simulink model "CCMHfaMotor"  (R2022b)
settings: Solver=FixedStepDiscrete  StartTime=0.0  StopTime=10.0  FixedStep=0.01  RelTol=1e-3
model workspace (MAT data in the model file):
  HfaWakeUpFlag : Simulink.Signal (value not decoded)
  NvMBlockHfaMtrCalData : Simulink.Signal (value not decoded)
  NvMBlockHfaMtrCalDataInit : Simulink.Parameter (value not decoded)
symbols that are objects in the model workspace (value not decoded): NvMBlockHfaMtrCalDataInit
undefined symbols (not defined in the file or in its folder): ComChlType, DioChannelType, HFAChlType, LinFrameType, NetworkChlType, NvMBlockId, P_DioLevelType, P_LinIfSchHandleType, P_Std_ReturnType, PowerChlType
(part 4 of 4)

== system CCMHfaMotor/HfaNvmService ==
  HfaNvmService_ClerarNvmMotorData_sys : Subsystem

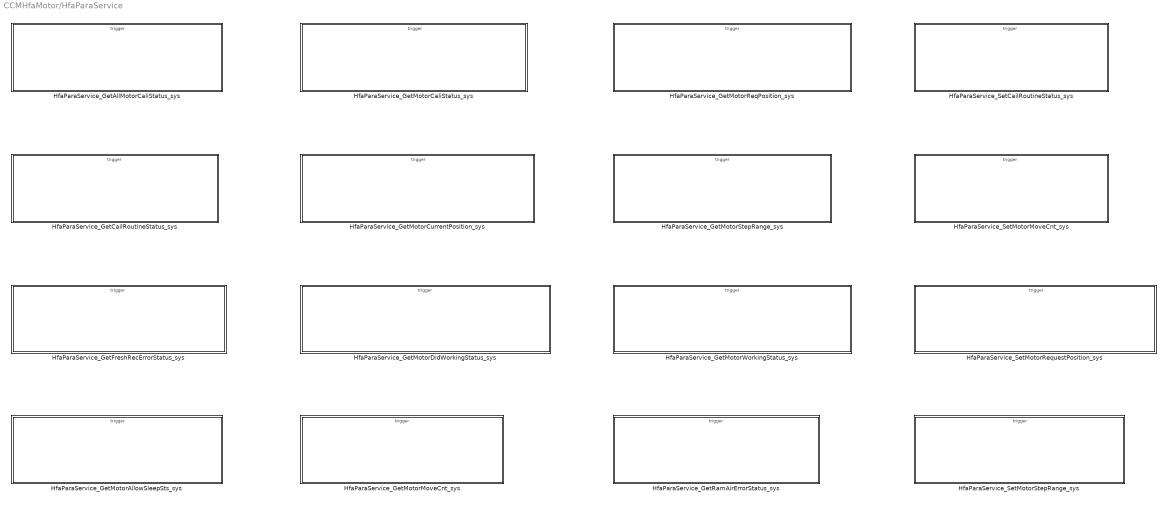
== system CCMHfaMotor/HfaParaService ==
  HfaParaService_GetAllMotorCaliStatus_sys : Subsystem
  HfaParaService_GetCailRoutineStatus_sys : Subsystem
  HfaParaService_GetFreshRecErrorStatus_sys : Subsystem
  HfaParaService_GetMotorAllowSleepSts_sys : Subsystem
  HfaParaService_GetMotorCaliStatus_sys : Subsystem
  HfaParaService_GetMotorCurrentPosition_sys : Subsystem
  HfaParaService_GetMotorDidWorkingStatus_sys : Subsystem
  HfaParaService_GetMotorMoveCnt_sys : Subsystem
  HfaParaService_GetMotorReqPosition_sys : Subsystem
  HfaParaService_GetMotorStepRange_sys : Subsystem
  HfaParaService_GetMotorWorkingStatus_sys : Subsystem
  HfaParaService_GetRamAirErrorStatus_sys : Subsystem
  HfaParaService_SetCailRoutineStatus_sys : Subsystem
  HfaParaService_SetMotorMoveCnt_sys : Subsystem
  HfaParaService_SetMotorRequestPosition_sys : Subsystem
  HfaParaService_SetMotorStepRange_sys : Subsystem

== system CCMHfaMotor/HfaRIDService ==
  HfaRIDService_GetAutoAddreRoutineSts_sys : Subsystem
  HfaRIDService_GetMotorAutoAddreSts_sys : Subsystem
  HfaRIDService_SetAutoAddreRoutineSts_sys : Subsystem
  HfaRIDService_SetMotorAutoAddre_sys : Subsystem

== system CCMHfaMotor/HfaCaliService/HfaCaliService_SetAllCalibrate_sys ==
  function : TriggerPort  TriggerType=function-call  IsSimulinkFunction=on  FunctionName=HfaCaliService_SetAllCalibrate  VariantControl=(inherit)
  Ground_1 : Ground
  PA_NvMBlockHfaMtrCalDataInit : Constant  Value=NvMBlockHfaMtrCalDataInit
  Terminator_1 : Terminator
  ERR : ArgOut  OutDataTypeStr=Std_ReturnType  ArgumentName=ERR
  wires:
  PA_NvMBlockHfaMtrCalDataInit/1 -> Terminator_1/1
  Ground_1/1 -> ERR/1

== system CCMHfaMotor/HfaCaliService/HfaCaliService_SetAllParaInit_sys ==
  function : TriggerPort  TriggerType=function-call  IsSimulinkFunction=on  FunctionName=HfaCaliService_SetAllParaInit  VariantControl=(inherit)
  Ground_1 : Ground
  PA_NvMBlockHfaMtrCalDataInit : Constant  Value=NvMBlockHfaMtrCalDataInit
  Terminator_1 : Terminator
  ERR : ArgOut  OutDataTypeStr=Std_ReturnType  ArgumentName=ERR
  wires:
  PA_NvMBlockHfaMtrCalDataInit/1 -> Terminator_1/1
  Ground_1/1 -> ERR/1

== system CCMHfaMotor/HfaCaliService/HfaCaliService_SetMotorCalibrate_sys ==
  function : TriggerPort  TriggerType=function-call  IsSimulinkFunction=on  FunctionName=HfaCaliService_SetMotorCalibrate  VariantControl=(inherit)
  Ground_1 : Ground
  PA_NvMBlockHfaMtrCalDataInit : Constant  Value=NvMBlockHfaMtrCalDataInit
  Terminator_1 : Terminator
  Terminator_2 : Terminator
  Channel : ArgIn  OutDataTypeStr=Enum: HFAChlType  ArgumentName=Channel
  ERR : ArgOut  OutDataTypeStr=Std_ReturnType  ArgumentName=ERR
  wires:
  PA_NvMBlockHfaMtrCalDataInit/1 -> Terminator_1/1
  Channel/1 -> Terminator_2/1
  Ground_1/1 -> ERR/1

== system CCMHfaMotor/HfaCaliService/HfaCaliService_SetMotorParaInit_sys ==
  function : TriggerPort  TriggerType=function-call  IsSimulinkFunction=on  FunctionName=HfaCaliService_SetMotorParaInit  VariantControl=(inherit)
  Ground_1 : Ground
  PA_NvMBlockHfaMtrCalDataInit : Constant  Value=NvMBlockHfaMtrCalDataInit
  Terminator_1 : Terminator
  Terminator_2 : Terminator
  Channel : ArgIn  OutDataTypeStr=Enum: HFAChlType  ArgumentName=Channel
  ERR : ArgOut  OutDataTypeStr=Std_ReturnType  ArgumentName=ERR
  wires:
  PA_NvMBlockHfaMtrCalDataInit/1 -> Terminator_1/1
  Channel/1 -> Terminator_2/1
  Ground_1/1 -> ERR/1

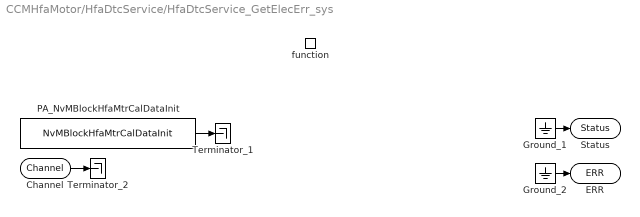
== system CCMHfaMotor/HfaDtcService/HfaDtcService_GetElecErr_sys ==
  function : TriggerPort  TriggerType=function-call  IsSimulinkFunction=on  FunctionName=HfaDtcService_GetElecErr  VariantControl=(inherit)
  Ground_1 : Ground
  Ground_2 : Ground
  PA_NvMBlockHfaMtrCalDataInit : Constant  Value=NvMBlockHfaMtrCalDataInit
  Terminator_1 : Terminator
  Terminator_2 : Terminator
  Channel : ArgIn  OutDataTypeStr=Enum: HFAChlType  ArgumentName=Channel
  Status : ArgOut  OutDataTypeStr=UInt8  ArgumentName=Status
  ERR : ArgOut  Port=2  OutDataTypeStr=Std_ReturnType  ArgumentName=ERR
  wires:
  PA_NvMBlockHfaMtrCalDataInit/1 -> Terminator_1/1
  Channel/1 -> Terminator_2/1
  Ground_1/1 -> Status/1
  Ground_2/1 -> ERR/1

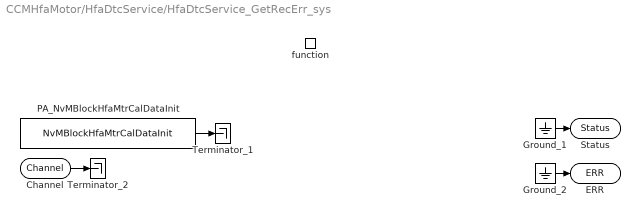
== system CCMHfaMotor/HfaDtcService/HfaDtcService_GetRecErr_sys ==
  function : TriggerPort  TriggerType=function-call  IsSimulinkFunction=on  FunctionName=HfaDtcService_GetRecErr  VariantControl=(inherit)
  Ground_1 : Ground
  Ground_2 : Ground
  PA_NvMBlockHfaMtrCalDataInit : Constant  Value=NvMBlockHfaMtrCalDataInit
  Terminator_1 : Terminator
  Terminator_2 : Terminator
  Channel : ArgIn  OutDataTypeStr=Enum: HFAChlType  ArgumentName=Channel
  Status : ArgOut  OutDataTypeStr=UInt8  ArgumentName=Status
  ERR : ArgOut  Port=2  OutDataTypeStr=Std_ReturnType  ArgumentName=ERR
  wires:
  PA_NvMBlockHfaMtrCalDataInit/1 -> Terminator_1/1
  Channel/1 -> Terminator_2/1
  Ground_1/1 -> Status/1
  Ground_2/1 -> ERR/1

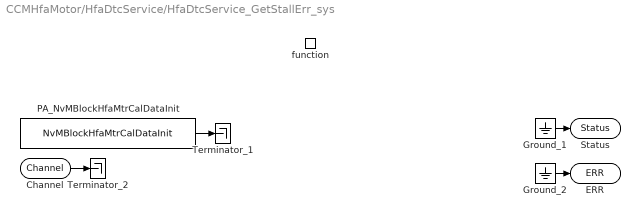
== system CCMHfaMotor/HfaDtcService/HfaDtcService_GetStallErr_sys ==
  function : TriggerPort  TriggerType=function-call  IsSimulinkFunction=on  FunctionName=HfaDtcService_GetStallErr  VariantControl=(inherit)
  Ground_1 : Ground
  Ground_2 : Ground
  PA_NvMBlockHfaMtrCalDataInit : Constant  Value=NvMBlockHfaMtrCalDataInit
  Terminator_1 : Terminator
  Terminator_2 : Terminator
  Channel : ArgIn  OutDataTypeStr=Enum: HFAChlType  ArgumentName=Channel
  Status : ArgOut  OutDataTypeStr=UInt8  ArgumentName=Status
  ERR : ArgOut  Port=2  OutDataTypeStr=Std_ReturnType  ArgumentName=ERR
  wires:
  PA_NvMBlockHfaMtrCalDataInit/1 -> Terminator_1/1
  Channel/1 -> Terminator_2/1
  Ground_1/1 -> Status/1
  Ground_2/1 -> ERR/1

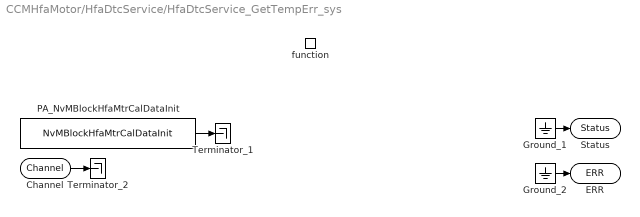
== system CCMHfaMotor/HfaDtcService/HfaDtcService_GetTempErr_sys ==
  function : TriggerPort  TriggerType=function-call  IsSimulinkFunction=on  FunctionName=HfaDtcService_GetTempErr  VariantControl=(inherit)
  Ground_1 : Ground
  Ground_2 : Ground
  PA_NvMBlockHfaMtrCalDataInit : Constant  Value=NvMBlockHfaMtrCalDataInit
  Terminator_1 : Terminator
  Terminator_2 : Terminator
  Channel : ArgIn  OutDataTypeStr=Enum: HFAChlType  ArgumentName=Channel
  Status : ArgOut  OutDataTypeStr=UInt8  ArgumentName=Status
  ERR : ArgOut  Port=2  OutDataTypeStr=Std_ReturnType  ArgumentName=ERR
  wires:
  PA_NvMBlockHfaMtrCalDataInit/1 -> Terminator_1/1
  Channel/1 -> Terminator_2/1
  Ground_1/1 -> Status/1
  Ground_2/1 -> ERR/1

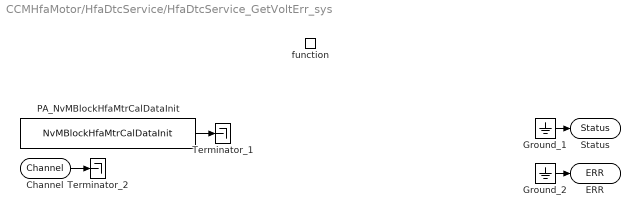
== system CCMHfaMotor/HfaDtcService/HfaDtcService_GetVoltErr_sys ==
  function : TriggerPort  TriggerType=function-call  IsSimulinkFunction=on  FunctionName=HfaDtcService_GetVoltErr  VariantControl=(inherit)
  Ground_1 : Ground
  Ground_2 : Ground
  PA_NvMBlockHfaMtrCalDataInit : Constant  Value=NvMBlockHfaMtrCalDataInit
  Terminator_1 : Terminator
  Terminator_2 : Terminator
  Channel : ArgIn  OutDataTypeStr=Enum: HFAChlType  ArgumentName=Channel
  Status : ArgOut  OutDataTypeStr=UInt8  ArgumentName=Status
  ERR : ArgOut  Port=2  OutDataTypeStr=Std_ReturnType  ArgumentName=ERR
  wires:
  PA_NvMBlockHfaMtrCalDataInit/1 -> Terminator_1/1
  Channel/1 -> Terminator_2/1
  Ground_1/1 -> Status/1
  Ground_2/1 -> ERR/1

== system CCMHfaMotor/HfaIOCtrlService/HfaIOCtrlService_FreezeMotorCurrentPosition_sys ==
  function : TriggerPort  TriggerType=function-call  IsSimulinkFunction=on  FunctionName=HfaIOCtrlService_FreezeMotorCurrentPosition  VariantControl=(inherit)
  Ground_1 : Ground
  PA_NvMBlockHfaMtrCalDataInit : Constant  Value=NvMBlockHfaMtrCalDataInit
  Terminator_1 : Terminator
  Terminator_2 : Terminator
  Channel : ArgIn  OutDataTypeStr=Enum: HFAChlType  ArgumentName=Channel
  ERR : ArgOut  OutDataTypeStr=Std_ReturnType  ArgumentName=ERR
  wires:
  PA_NvMBlockHfaMtrCalDataInit/1 -> Terminator_1/1
  Channel/1 -> Terminator_2/1
  Ground_1/1 -> ERR/1

== system CCMHfaMotor/HfaIOCtrlService/HfaIOCtrlService_MotorReturnToECU_sys ==
  function : TriggerPort  TriggerType=function-call  IsSimulinkFunction=on  FunctionName=HfaIOCtrlService_MotorReturnToECU  VariantControl=(inherit)
  Ground_1 : Ground
  PA_NvMBlockHfaMtrCalDataInit : Constant  Value=NvMBlockHfaMtrCalDataInit
  Terminator_1 : Terminator
  Terminator_2 : Terminator
  Channel : ArgIn  OutDataTypeStr=Enum: HFAChlType  ArgumentName=Channel
  ERR : ArgOut  OutDataTypeStr=Std_ReturnType  ArgumentName=ERR
  wires:
  PA_NvMBlockHfaMtrCalDataInit/1 -> Terminator_1/1
  Channel/1 -> Terminator_2/1
  Ground_1/1 -> ERR/1

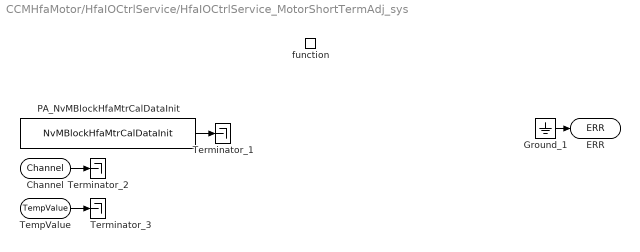
== system CCMHfaMotor/HfaIOCtrlService/HfaIOCtrlService_MotorShortTermAdj_sys ==
  function : TriggerPort  TriggerType=function-call  IsSimulinkFunction=on  FunctionName=HfaIOCtrlService_MotorShortTermAdj  VariantControl=(inherit)
  Ground_1 : Ground
  PA_NvMBlockHfaMtrCalDataInit : Constant  Value=NvMBlockHfaMtrCalDataInit
  Terminator_1 : Terminator
  Terminator_2 : Terminator
  Terminator_3 : Terminator
  Channel : ArgIn  OutDataTypeStr=Enum: HFAChlType  ArgumentName=Channel
  TempValue : ArgIn  Port=2  OutDataTypeStr=UInt16  ArgumentName=TempValue
  ERR : ArgOut  OutDataTypeStr=Std_ReturnType  ArgumentName=ERR
  wires:
  PA_NvMBlockHfaMtrCalDataInit/1 -> Terminator_1/1
  Channel/1 -> Terminator_2/1
  TempValue/1 -> Terminator_3/1
  Ground_1/1 -> ERR/1

== system CCMHfaMotor/HfaNvmService/HfaNvmService_ClerarNvmMotorData_sys ==
  function : TriggerPort  TriggerType=function-call  IsSimulinkFunction=on  FunctionName=HfaNvmService_ClerarNvmMotorData  VariantControl=(inherit)
  Ground_1 : Ground
  PA_NvMBlockHfaMtrCalDataInit : Constant  Value=NvMBlockHfaMtrCalDataInit
  Terminator_1 : Terminator
  ERR : ArgOut  OutDataTypeStr=Std_ReturnType  ArgumentName=ERR
  wires:
  PA_NvMBlockHfaMtrCalDataInit/1 -> Terminator_1/1
  Ground_1/1 -> ERR/1

== system CCMHfaMotor/HfaParaService/HfaParaService_GetAllMotorCaliStatus_sys ==
  function : TriggerPort  TriggerType=function-call  IsSimulinkFunction=on  FunctionName=HfaParaService_GetAllMotorCaliStatus  VariantControl=(inherit)
  Ground_1 : Ground
  Ground_2 : Ground
  PA_NvMBlockHfaMtrCalDataInit : Constant  Value=NvMBlockHfaMtrCalDataInit
  Terminator_1 : Terminator
  Status : ArgOut  OutDataTypeStr=UInt8  ArgumentName=Status
  ERR : ArgOut  Port=2  OutDataTypeStr=Std_ReturnType  ArgumentName=ERR
  wires:
  PA_NvMBlockHfaMtrCalDataInit/1 -> Terminator_1/1
  Ground_1/1 -> Status/1
  Ground_2/1 -> ERR/1

== system CCMHfaMotor/HfaParaService/HfaParaService_GetCailRoutineStatus_sys ==
  function : TriggerPort  TriggerType=function-call  IsSimulinkFunction=on  FunctionName=HfaParaService_GetCailRoutineStatus  VariantControl=(inherit)
  Ground_1 : Ground
  Ground_2 : Ground
  PA_NvMBlockHfaMtrCalDataInit : Constant  Value=NvMBlockHfaMtrCalDataInit
  Terminator_1 : Terminator
  Status : ArgOut  OutDataTypeStr=Enum: MtrRoutStaEnum  ArgumentName=Status
  ERR : ArgOut  Port=2  OutDataTypeStr=Std_ReturnType  ArgumentName=ERR
  wires:
  PA_NvMBlockHfaMtrCalDataInit/1 -> Terminator_1/1
  Ground_1/1 -> Status/1
  Ground_2/1 -> ERR/1

== system CCMHfaMotor/HfaParaService/HfaParaService_GetFreshRecErrorStatus_sys ==
  function : TriggerPort  TriggerType=function-call  IsSimulinkFunction=on  FunctionName=HfaParaService_GetFreshRecErrorStatus  VariantControl=(inherit)
  Ground_1 : Ground
  Ground_2 : Ground
  PA_NvMBlockHfaMtrCalDataInit : Constant  Value=NvMBlockHfaMtrCalDataInit
  Terminator_1 : Terminator
  Status : ArgOut  OutDataTypeStr=UInt8  ArgumentName=Status
  ERR : ArgOut  Port=2  OutDataTypeStr=Std_ReturnType  ArgumentName=ERR
  wires:
  PA_NvMBlockHfaMtrCalDataInit/1 -> Terminator_1/1
  Ground_1/1 -> Status/1
  Ground_2/1 -> ERR/1

== system CCMHfaMotor/HfaParaService/HfaParaService_GetMotorAllowSleepSts_sys ==
  function : TriggerPort  TriggerType=function-call  IsSimulinkFunction=on  FunctionName=HfaParaService_GetMotorAllowSleepSts  VariantControl=(inherit)
  Ground_1 : Ground
  Ground_2 : Ground
  PA_NvMBlockHfaMtrCalDataInit : Constant  Value=NvMBlockHfaMtrCalDataInit
  Terminator_1 : Terminator
  Status : ArgOut  OutDataTypeStr=UInt8  ArgumentName=Status
  ERR : ArgOut  Port=2  OutDataTypeStr=Std_ReturnType  ArgumentName=ERR
  wires:
  PA_NvMBlockHfaMtrCalDataInit/1 -> Terminator_1/1
  Ground_1/1 -> Status/1
  Ground_2/1 -> ERR/1

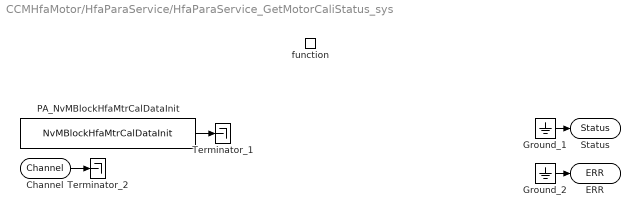
== system CCMHfaMotor/HfaParaService/HfaParaService_GetMotorCaliStatus_sys ==
  function : TriggerPort  TriggerType=function-call  IsSimulinkFunction=on  FunctionName=HfaParaService_GetMotorCaliStatus  VariantControl=(inherit)
  Ground_1 : Ground
  Ground_2 : Ground
  PA_NvMBlockHfaMtrCalDataInit : Constant  Value=NvMBlockHfaMtrCalDataInit
  Terminator_1 : Terminator
  Terminator_2 : Terminator
  Channel : ArgIn  OutDataTypeStr=Enum: HFAChlType  ArgumentName=Channel
  Status : ArgOut  OutDataTypeStr=UInt8  ArgumentName=Status
  ERR : ArgOut  Port=2  OutDataTypeStr=Std_ReturnType  ArgumentName=ERR
  wires:
  PA_NvMBlockHfaMtrCalDataInit/1 -> Terminator_1/1
  Channel/1 -> Terminator_2/1
  Ground_1/1 -> Status/1
  Ground_2/1 -> ERR/1

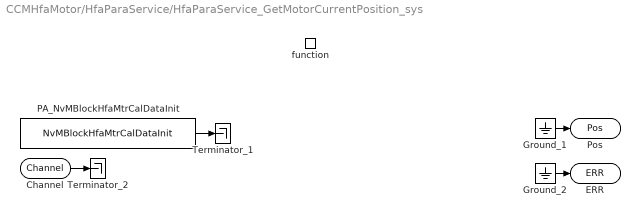
== system CCMHfaMotor/HfaParaService/HfaParaService_GetMotorCurrentPosition_sys ==
  function : TriggerPort  TriggerType=function-call  IsSimulinkFunction=on  FunctionName=HfaParaService_GetMotorCurrentPosition  VariantControl=(inherit)
  Ground_1 : Ground
  Ground_2 : Ground
  PA_NvMBlockHfaMtrCalDataInit : Constant  Value=NvMBlockHfaMtrCalDataInit
  Terminator_1 : Terminator
  Terminator_2 : Terminator
  Channel : ArgIn  OutDataTypeStr=Enum: HFAChlType  ArgumentName=Channel
  Pos : ArgOut  OutDataTypeStr=UInt16  ArgumentName=Pos
  ERR : ArgOut  Port=2  OutDataTypeStr=Std_ReturnType  ArgumentName=ERR
  wires:
  PA_NvMBlockHfaMtrCalDataInit/1 -> Terminator_1/1
  Channel/1 -> Terminator_2/1
  Ground_1/1 -> Pos/1
  Ground_2/1 -> ERR/1

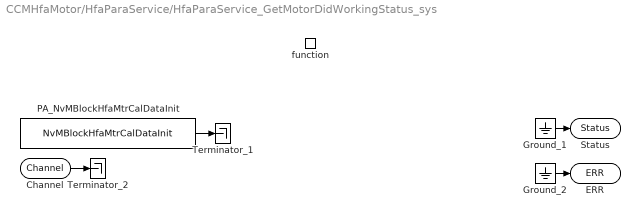
== system CCMHfaMotor/HfaParaService/HfaParaService_GetMotorDidWorkingStatus_sys ==
  function : TriggerPort  TriggerType=function-call  IsSimulinkFunction=on  FunctionName=HfaParaService_GetMotorDidWorkingStatus  VariantControl=(inherit)
  Ground_1 : Ground
  Ground_2 : Ground
  PA_NvMBlockHfaMtrCalDataInit : Constant  Value=NvMBlockHfaMtrCalDataInit
  Terminator_1 : Terminator
  Terminator_2 : Terminator
  Channel : ArgIn  OutDataTypeStr=Enum: HFAChlType  ArgumentName=Channel
  Status : ArgOut  OutDataTypeStr=UInt8  ArgumentName=Status
  ERR : ArgOut  Port=2  OutDataTypeStr=Std_ReturnType  ArgumentName=ERR
  wires:
  PA_NvMBlockHfaMtrCalDataInit/1 -> Terminator_1/1
  Channel/1 -> Terminator_2/1
  Ground_1/1 -> Status/1
  Ground_2/1 -> ERR/1

== system CCMHfaMotor/HfaParaService/HfaParaService_GetMotorMoveCnt_sys ==
  function : TriggerPort  TriggerType=function-call  IsSimulinkFunction=on  FunctionName=HfaParaService_GetMotorMoveCnt  VariantControl=(inherit)
  Ground_1 : Ground
  Ground_2 : Ground
  PA_NvMBlockHfaMtrCalDataInit : Constant  Value=NvMBlockHfaMtrCalDataInit
  Terminator_1 : Terminator
  Terminator_2 : Terminator
  Channel : ArgIn  OutDataTypeStr=Enum: HFAChlType  ArgumentName=Channel
  Cnt : ArgOut  OutDataTypeStr=UInt32  ArgumentName=Cnt
  ERR : ArgOut  Port=2  OutDataTypeStr=Std_ReturnType  ArgumentName=ERR
  wires:
  PA_NvMBlockHfaMtrCalDataInit/1 -> Terminator_1/1
  Channel/1 -> Terminator_2/1
  Ground_1/1 -> Cnt/1
  Ground_2/1 -> ERR/1

== system CCMHfaMotor/HfaParaService/HfaParaService_GetMotorReqPosition_sys ==
  function : TriggerPort  TriggerType=function-call  IsSimulinkFunction=on  FunctionName=HfaParaService_GetMotorReqPosition  VariantControl=(inherit)
  Ground_1 : Ground
  Ground_2 : Ground
  PA_NvMBlockHfaMtrCalDataInit : Constant  Value=NvMBlockHfaMtrCalDataInit
  Terminator_1 : Terminator
  Terminator_2 : Terminator
  Channel : ArgIn  OutDataTypeStr=Enum: HFAChlType  ArgumentName=Channel
  Position : ArgOut  OutDataTypeStr=UInt16  ArgumentName=Position
  ERR : ArgOut  Port=2  OutDataTypeStr=Std_ReturnType  ArgumentName=ERR
  wires:
  PA_NvMBlockHfaMtrCalDataInit/1 -> Terminator_1/1
  Channel/1 -> Terminator_2/1
  Ground_1/1 -> Position/1
  Ground_2/1 -> ERR/1

== system CCMHfaMotor/HfaParaService/HfaParaService_GetMotorStepRange_sys ==
  function : TriggerPort  TriggerType=function-call  IsSimulinkFunction=on  FunctionName=HfaParaService_GetMotorStepRange  VariantControl=(inherit)
  Ground_1 : Ground
  Ground_2 : Ground
  PA_NvMBlockHfaMtrCalDataInit : Constant  Value=NvMBlockHfaMtrCalDataInit
  Terminator_1 : Terminator
  Terminator_2 : Terminator
  Channel : ArgIn  OutDataTypeStr=Enum: HFAChlType  ArgumentName=Channel
  Range : ArgOut  OutDataTypeStr=UInt16  ArgumentName=Range
  ERR : ArgOut  Port=2  OutDataTypeStr=Std_ReturnType  ArgumentName=ERR
  wires:
  PA_NvMBlockHfaMtrCalDataInit/1 -> Terminator_1/1
  Channel/1 -> Terminator_2/1
  Ground_1/1 -> Range/1
  Ground_2/1 -> ERR/1

== system CCMHfaMotor/HfaParaService/HfaParaService_GetMotorWorkingStatus_sys ==
  function : TriggerPort  TriggerType=function-call  IsSimulinkFunction=on  FunctionName=HfaParaService_GetMotorWorkingStatus  VariantControl=(inherit)
  Ground_1 : Ground
  Ground_2 : Ground
  PA_NvMBlockHfaMtrCalDataInit : Constant  Value=NvMBlockHfaMtrCalDataInit
  Terminator_1 : Terminator
  Terminator_2 : Terminator
  Channel : ArgIn  OutDataTypeStr=Enum: HFAChlType  ArgumentName=Channel
  Status : ArgOut  OutDataTypeStr=UInt8  ArgumentName=Status
  ERR : ArgOut  Port=2  OutDataTypeStr=Std_ReturnType  ArgumentName=ERR
  wires:
  PA_NvMBlockHfaMtrCalDataInit/1 -> Terminator_1/1
  Channel/1 -> Terminator_2/1
  Ground_1/1 -> Status/1
  Ground_2/1 -> ERR/1

== system CCMHfaMotor/HfaParaService/HfaParaService_GetRamAirErrorStatus_sys ==
  function : TriggerPort  TriggerType=function-call  IsSimulinkFunction=on  FunctionName=HfaParaService_GetRamAirErrorStatus  VariantControl=(inherit)
  Ground_1 : Ground
  Ground_2 : Ground
  PA_NvMBlockHfaMtrCalDataInit : Constant  Value=NvMBlockHfaMtrCalDataInit
  Terminator_1 : Terminator
  Status : ArgOut  OutDataTypeStr=UInt8  ArgumentName=Status
  ERR : ArgOut  Port=2  OutDataTypeStr=Std_ReturnType  ArgumentName=ERR
  wires:
  PA_NvMBlockHfaMtrCalDataInit/1 -> Terminator_1/1
  Ground_1/1 -> Status/1
  Ground_2/1 -> ERR/1

== system CCMHfaMotor/HfaParaService/HfaParaService_SetCailRoutineStatus_sys ==
  function : TriggerPort  TriggerType=function-call  IsSimulinkFunction=on  FunctionName=HfaParaService_SetCailRoutineStatus  VariantControl=(inherit)
  Ground_1 : Ground
  PA_NvMBlockHfaMtrCalDataInit : Constant  Value=NvMBlockHfaMtrCalDataInit
  Terminator_1 : Terminator
  Terminator_2 : Terminator
  Status : ArgIn  OutDataTypeStr=Enum: MtrRoutStaEnum  ArgumentName=Status
  ERR : ArgOut  OutDataTypeStr=Std_ReturnType  ArgumentName=ERR
  wires:
  PA_NvMBlockHfaMtrCalDataInit/1 -> Terminator_1/1
  Status/1 -> Terminator_2/1
  Ground_1/1 -> ERR/1

== system CCMHfaMotor/HfaParaService/HfaParaService_SetMotorMoveCnt_sys ==
  function : TriggerPort  TriggerType=function-call  IsSimulinkFunction=on  FunctionName=HfaParaService_SetMotorMoveCnt  VariantControl=(inherit)
  Ground_1 : Ground
  PA_NvMBlockHfaMtrCalDataInit : Constant  Value=NvMBlockHfaMtrCalDataInit
  Terminator_1 : Terminator
  Terminator_2 : Terminator
  Terminator_3 : Terminator
  Channel : ArgIn  OutDataTypeStr=Enum: HFAChlType  ArgumentName=Channel
  Cnt : ArgIn  Port=2  OutDataTypeStr=UInt32  ArgumentName=Cnt
  ERR : ArgOut  OutDataTypeStr=Std_ReturnType  ArgumentName=ERR
  wires:
  PA_NvMBlockHfaMtrCalDataInit/1 -> Terminator_1/1
  Channel/1 -> Terminator_2/1
  Cnt/1 -> Terminator_3/1
  Ground_1/1 -> ERR/1

== system CCMHfaMotor/HfaParaService/HfaParaService_SetMotorRequestPosition_sys ==
  function : TriggerPort  TriggerType=function-call  IsSimulinkFunction=on  FunctionName=HfaParaService_SetMotorRequestPosition  VariantControl=(inherit)
  Ground_1 : Ground
  PA_NvMBlockHfaMtrCalDataInit : Constant  Value=NvMBlockHfaMtrCalDataInit
  Terminator_1 : Terminator
  Terminator_2 : Terminator
  Terminator_3 : Terminator
  Channel : ArgIn  OutDataTypeStr=Enum: HFAChlType  ArgumentName=Channel
  Present : ArgIn  Port=2  OutDataTypeStr=UInt16  ArgumentName=Present
  ERR : ArgOut  OutDataTypeStr=Std_ReturnType  ArgumentName=ERR
  wires:
  PA_NvMBlockHfaMtrCalDataInit/1 -> Terminator_1/1
  Channel/1 -> Terminator_2/1
  Present/1 -> Terminator_3/1
  Ground_1/1 -> ERR/1

== system CCMHfaMotor/HfaParaService/HfaParaService_SetMotorStepRange_sys ==
  function : TriggerPort  TriggerType=function-call  IsSimulinkFunction=on  FunctionName=HfaParaService_SetMotorStepRange  VariantControl=(inherit)
  Ground_1 : Ground
  PA_NvMBlockHfaMtrCalDataInit : Constant  Value=NvMBlockHfaMtrCalDataInit
  Terminator_1 : Terminator
  Terminator_2 : Terminator
  Terminator_3 : Terminator
  Channel : ArgIn  OutDataTypeStr=Enum: HFAChlType  ArgumentName=Channel
  Range : ArgIn  Port=2  OutDataTypeStr=UInt16  ArgumentName=Range
  ERR : ArgOut  OutDataTypeStr=Std_ReturnType  ArgumentName=ERR
  wires:
  PA_NvMBlockHfaMtrCalDataInit/1 -> Terminator_1/1
  Channel/1 -> Terminator_2/1
  Range/1 -> Terminator_3/1
  Ground_1/1 -> ERR/1

== system CCMHfaMotor/HfaRIDService/HfaRIDService_GetAutoAddreRoutineSts_sys ==
  function : TriggerPort  TriggerType=function-call  IsSimulinkFunction=on  FunctionName=HfaRIDService_GetAutoAddreRoutineSts  VariantControl=(inherit)
  Ground_1 : Ground
  Ground_2 : Ground
  PA_NvMBlockHfaMtrCalDataInit : Constant  Value=NvMBlockHfaMtrCalDataInit
  Terminator_1 : Terminator
  Status : ArgOut  OutDataTypeStr=Enum: MtrRoutStaEnum  ArgumentName=Status
  ERR : ArgOut  Port=2  OutDataTypeStr=Std_ReturnType  ArgumentName=ERR
  wires:
  PA_NvMBlockHfaMtrCalDataInit/1 -> Terminator_1/1
  Ground_1/1 -> Status/1
  Ground_2/1 -> ERR/1

== system CCMHfaMotor/HfaRIDService/HfaRIDService_GetMotorAutoAddreSts_sys ==
  function : TriggerPort  TriggerType=function-call  IsSimulinkFunction=on  FunctionName=HfaRIDService_GetMotorAutoAddreSts  VariantControl=(inherit)
  Ground_1 : Ground
  Ground_2 : Ground
  PA_NvMBlockHfaMtrCalDataInit : Constant  Value=NvMBlockHfaMtrCalDataInit
  Terminator_1 : Terminator
  Status : ArgOut  OutDataTypeStr=UInt8  ArgumentName=Status
  ERR : ArgOut  Port=2  OutDataTypeStr=Std_ReturnType  ArgumentName=ERR
  wires:
  PA_NvMBlockHfaMtrCalDataInit/1 -> Terminator_1/1
  Ground_1/1 -> Status/1
  Ground_2/1 -> ERR/1

== system CCMHfaMotor/HfaRIDService/HfaRIDService_SetAutoAddreRoutineSts_sys ==
  function : TriggerPort  TriggerType=function-call  IsSimulinkFunction=on  FunctionName=HfaRIDService_SetAutoAddreRoutineSts  VariantControl=(inherit)
  Ground_1 : Ground
  PA_NvMBlockHfaMtrCalDataInit : Constant  Value=NvMBlockHfaMtrCalDataInit
  Terminator_1 : Terminator
  Terminator_2 : Terminator
  Status : ArgIn  OutDataTypeStr=Enum: MtrRoutStaEnum  ArgumentName=Status
  ERR : ArgOut  OutDataTypeStr=Std_ReturnType  ArgumentName=ERR
  wires:
  PA_NvMBlockHfaMtrCalDataInit/1 -> Terminator_1/1
  Status/1 -> Terminator_2/1
  Ground_1/1 -> ERR/1

== system CCMHfaMotor/HfaRIDService/HfaRIDService_SetMotorAutoAddre_sys ==
  function : TriggerPort  TriggerType=function-call  IsSimulinkFunction=on  FunctionName=HfaRIDService_SetMotorAutoAddre  VariantControl=(inherit)
  Ground_1 : Ground
  PA_NvMBlockHfaMtrCalDataInit : Constant  Value=NvMBlockHfaMtrCalDataInit
  Terminator_1 : Terminator
  ERR : ArgOut  OutDataTypeStr=Std_ReturnType  ArgumentName=ERR
  wires:
  PA_NvMBlockHfaMtrCalDataInit/1 -> Terminator_1/1
  Ground_1/1 -> ERR/1
